simulink model "ROMmodel_C4_C5"  (R2023a)
settings: Solver=ode15s  StartTime=0.0  StopTime=1  RelTol=1e-3
model workspace (MAT data in the model file):
  AbdFlex : object (value not decoded)
  C_IVD = 0.05
  E_AbdFlex = 0.126
  E_IVD1 = 2.1
  E_IVD2 = 2.2
  E_IVD3 = 1.1
  E_IVD4 = 1.7
  E_IVD5 = 3.2
  E_IVD_Optimization : sldodialogs.data.SessionData (value not decoded)
  E_TLFflex = 18.8
  FollowerLoad = 0
  IAP_Pa = 0
  IAP_activation_AbdFlex = 1
  ISL_color = [1 1 1]
  ITL_color = [1 1 1]
  IVD1 : object (value not decoded)
  IVD2 : object (value not decoded)
  IVD3 : object (value not decoded)
  IVD4 : object (value not decoded)
  IVD5 : object (value not decoded)
  Left_EO = 0
  Left_IO = 0
  Left_Lat = 18
  Left_LongThor = 0
  Left_MF = 0
  Left_Psoas = 0
  Left_RA = 0
  Moment_AR = 0
  Moment_FE = 7.5
  Moment_LB = 0
  ROM_draft1_sdosession : sldodialogs.data.SessionData (value not decoded)
  ROM_draft3_sdosession : sldodialogs.data.SessionData (value not decoded)
  Right_EO = 0
  Right_IO = 0
  Right_Lat = 18
  Right_LongThor = 0
  Right_MF = 0
  Right_Psoas = 0
  Right_RA = 0
  SSL_color = [1 1 1]
  TLFflex : object (value not decoded)
  TLFflex2 : object (value not decoded)
  eo_color = [1 0 0]
  io_color = [0 1 0]
  latissimus_color = [0 0.5 1]
  ligament_radius = 0.5
  longissimus_color = [1 0 1]
  multifidus_color = [0 1 1]
  muscle_radius = 1
  psoas_color = [0 0 1]
  ra_color = [1 1 0.5]
libraries: checkpacklib x11, simulink_extras x9, simulink x2
settings Model: Creator=siril  LastModifiedBy=siril
settings DataIOCC: SaveFormat=StructureWithTime  ReturnWorkspaceOutputsName=SDOSimTest_Log
settings DebuggingCC: UniqueDataStoreMsg=error
settings SimscapeCC: SimscapeLogType=all

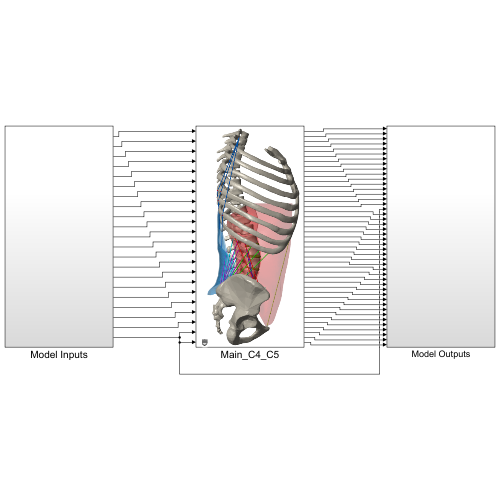
[thumbnail: Simulink's stored preview of the top-level diagram]
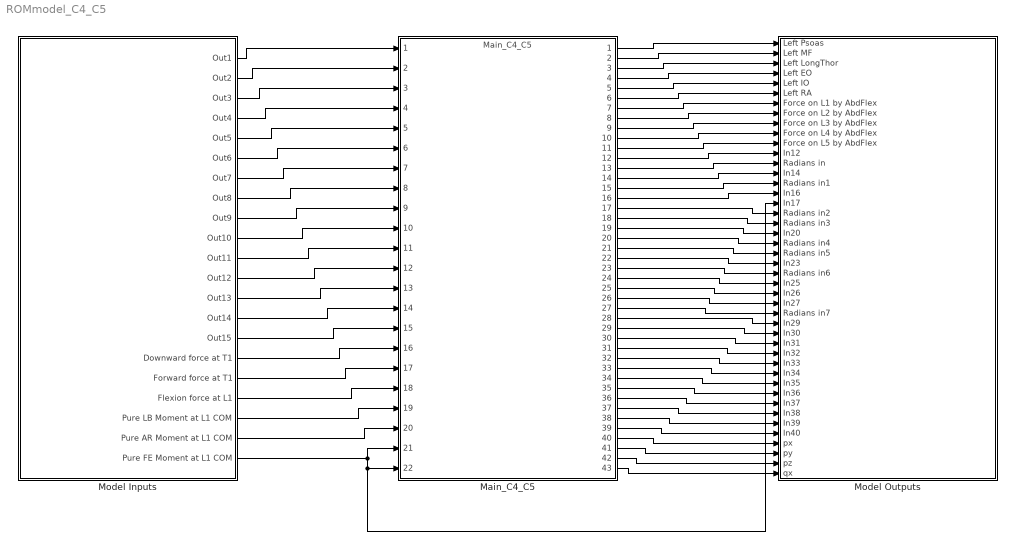
== system ROMmodel_C4_C5 ==
  Main_C4_C5 : ModelReference  ModelNameDialog=Main_C4_C5.slxp  ModelReferenceVersion=1.133  CopyOfModelProtected=on
      mask Display: image('$imagefile'); % Use "Edit Image" on toolstrip to change.
  Model Inputs : Subsystem
  Model Outputs : Subsystem
  wires:
  Main_C4_C5/32 -> Model Outputs/33(In33)
  Main_C4_C5/31 -> Model Outputs/32(In32)
  Model Inputs/20(Pure AR Moment at L1 COM) -> Main_C4_C5/20
  Model Inputs/1(Out1) -> Main_C4_C5/1
  Model Inputs/7(Out7) -> Main_C4_C5/7
  Model Inputs/8(Out8) -> Main_C4_C5/8
  Model Inputs/3(Out3) -> Main_C4_C5/3
  Main_C4_C5/20 -> Model Outputs/21(Radians in4)
  Model Inputs/2(Out2) -> Main_C4_C5/2
  Main_C4_C5/24 -> Model Outputs/25(In25)
  Main_C4_C5/23 -> Model Outputs/24(Radians in6)
  Model Inputs/12(Out12) -> Main_C4_C5/12
  Model Inputs/4(Out4) -> Main_C4_C5/4
  Main_C4_C5/13 -> Model Outputs/13(Radians in)
  Model Inputs/5(Out5) -> Main_C4_C5/5
  Model Inputs/6(Out6) -> Main_C4_C5/6
  Model Inputs/9(Out9) -> Main_C4_C5/9
  Main_C4_C5/19 -> Model Outputs/20(In20)
  Model Inputs/10(Out10) -> Main_C4_C5/10
  Main_C4_C5/22 -> Model Outputs/23(In23)
  Model Inputs/11(Out11) -> Main_C4_C5/11
  Main_C4_C5/25 -> Model Outputs/26(In26)
  Model Inputs/14(Out14) -> Main_C4_C5/14
  Model Inputs/13(Out13) -> Main_C4_C5/13
  Main_C4_C5/26 -> Model Outputs/27(In27)
  Model Inputs/15(Out15) -> Main_C4_C5/15
  Main_C4_C5/28 -> Model Outputs/29(In29)
  Main_C4_C5/27 -> Model Outputs/28(Radians in7)
  Model Inputs/16(Downward force at T1) -> Main_C4_C5/16
  Main_C4_C5/29 -> Model Outputs/30(In30)
  Model Inputs/18(Flexion force at L1) -> Main_C4_C5/18
  Model Inputs/17(Forward force at T1) -> Main_C4_C5/17
  Main_C4_C5/30 -> Model Outputs/31(In31)
  Model Inputs/19(Pure LB Moment at L1 COM) -> Main_C4_C5/19
  Main_C4_C5/33 -> Model Outputs/34(In34)
  Model Inputs/21(Pure FE Moment at L1 COM) -> Model Outputs/17(In17), Main_C4_C5/22, Main_C4_C5/21
  Main_C4_C5/1 -> Model Outputs/1(Left Psoas)
  Main_C4_C5/2 -> Model Outputs/2(Left MF)
  Main_C4_C5/3 -> Model Outputs/3(Left LongThor)
  Main_C4_C5/4 -> Model Outputs/4(Left EO)
  Main_C4_C5/5 -> Model Outputs/5(Left IO)
  Main_C4_C5/6 -> Model Outputs/6(Left RA)
  Main_C4_C5/7 -> Model Outputs/7(Force on L1 by AbdFlex)
  Main_C4_C5/8 -> Model Outputs/8(Force on L2 by AbdFlex)
  Main_C4_C5/9 -> Model Outputs/9(Force on L3 by AbdFlex)
  Main_C4_C5/10 -> Model Outputs/10(Force on L4 by AbdFlex)
  Main_C4_C5/11 -> Model Outputs/11(Force on L5 by AbdFlex)
  Main_C4_C5/12 -> Model Outputs/12(In12)
  Main_C4_C5/14 -> Model Outputs/14(In14)
  Main_C4_C5/15 -> Model Outputs/15(Radians in1)
  Main_C4_C5/16 -> Model Outputs/16(In16)
  Main_C4_C5/17 -> Model Outputs/18(Radians in2)
  Main_C4_C5/18 -> Model Outputs/19(Radians in3)
  Main_C4_C5/21 -> Model Outputs/22(Radians in5)
  Main_C4_C5/34 -> Model Outputs/35(In35)
  Main_C4_C5/36 -> Model Outputs/37(In37)
  Main_C4_C5/35 -> Model Outputs/36(In36)
  Main_C4_C5/37 -> Model Outputs/38(In38)
  Main_C4_C5/38 -> Model Outputs/39(In39)
  Main_C4_C5/40 -> Model Outputs/41(px)
  Main_C4_C5/39 -> Model Outputs/40(In40)
  Main_C4_C5/41 -> Model Outputs/42(py)
  Main_C4_C5/42 -> Model Outputs/43(pz)
  Main_C4_C5/43 -> Model Outputs/44(qx)

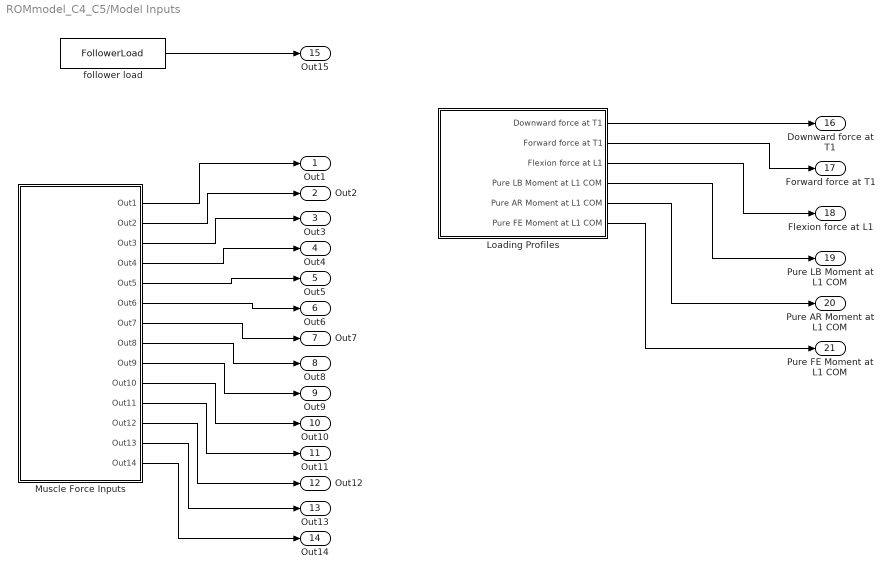
== system ROMmodel_C4_C5/Model Inputs ==
  Loading Profiles : Subsystem
  Muscle Force Inputs : Subsystem
  follower load : Constant  Value=FollowerLoad
  Out1 : Outport
  Out2 : Outport  Port=2
  Out3 : Outport  Port=3
  Out4 : Outport  Port=4
  Out5 : Outport  Port=5
  Out6 : Outport  Port=6
  Out7 : Outport  Port=7
  Out8 : Outport  Port=8
  Out9 : Outport  Port=9
  Out10 : Outport  Port=10
  Out11 : Outport  Port=11
  Out12 : Outport  Port=12
  Out13 : Outport  Port=13
  Out14 : Outport  Port=14
  Out15 : Outport  Port=15
  Downward force at T1 : Outport  Port=16
  Forward force at T1 : Outport  Port=17
  Flexion force at L1 : Outport  Port=18
  Pure LB Moment at L1 COM : Outport  Port=19
  Pure AR Moment at L1 COM : Outport  Port=20
  Pure FE Moment at L1 COM : Outport  Port=21
  wires:
  Muscle Force Inputs/12(Out12) -> Out12/1
  follower load/1 -> Out15/1
  Loading Profiles/6(Pure FE Moment at L1 COM) -> Pure FE Moment at L1 COM/1
  Loading Profiles/3(Flexion force at L1) -> Flexion force at L1/1
  Muscle Force Inputs/1(Out1) -> Out1/1
  Muscle Force Inputs/2(Out2) -> Out2/1
  Muscle Force Inputs/5(Out5) -> Out5/1
  Muscle Force Inputs/6(Out6) -> Out6/1
  Muscle Force Inputs/3(Out3) -> Out3/1
  Muscle Force Inputs/4(Out4) -> Out4/1
  Muscle Force Inputs/7(Out7) -> Out7/1
  Muscle Force Inputs/8(Out8) -> Out8/1
  Muscle Force Inputs/10(Out10) -> Out10/1
  Muscle Force Inputs/9(Out9) -> Out9/1
  Loading Profiles/4(Pure LB Moment at L1 COM) -> Pure LB Moment at L1 COM/1
  Muscle Force Inputs/11(Out11) -> Out11/1
  Muscle Force Inputs/13(Out13) -> Out13/1
  Muscle Force Inputs/14(Out14) -> Out14/1
  Loading Profiles/1(Downward force at T1) -> Downward force at T1/1
  Loading Profiles/2(Forward force at T1) -> Forward force at T1/1
  Loading Profiles/5(Pure AR Moment at L1 COM) -> Pure AR Moment at L1 COM/1

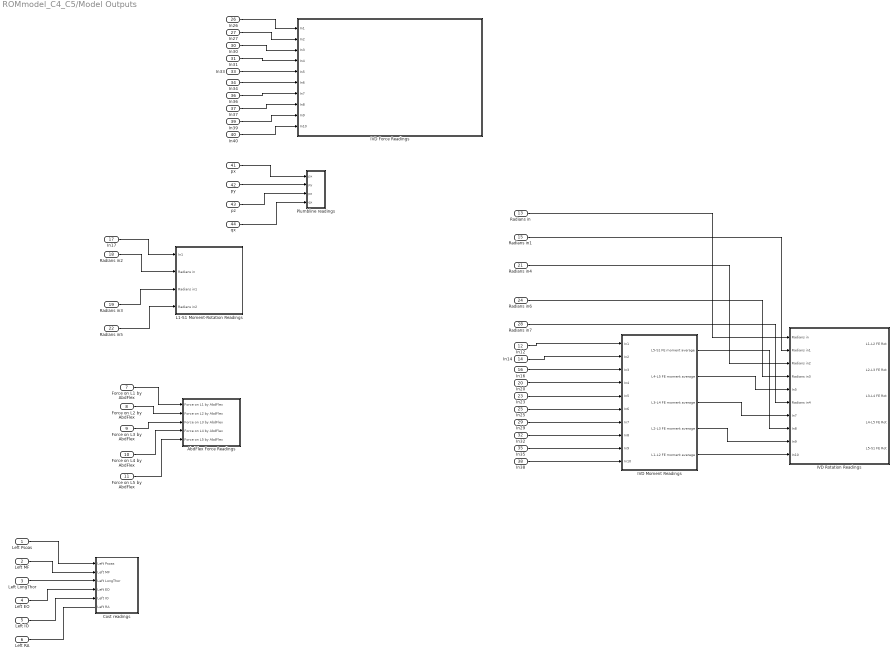
== system ROMmodel_C4_C5/Model Outputs ==
  Left Psoas : Inport
  Left MF : Inport  Port=2
  Left LongThor : Inport  Port=3
  Left EO : Inport  Port=4
  Left IO : Inport  Port=5
  Left RA : Inport  Port=6
  Force on L1 by AbdFlex : Inport  Port=7
  Force on L2 by AbdFlex : Inport  Port=8
  Force on L3 by AbdFlex : Inport  Port=9
  Force on L4 by AbdFlex : Inport  Port=10
  Force on L5 by AbdFlex : Inport  Port=11
  In12 : Inport  Port=12
  Radians in : Inport  Port=13
  In14 : Inport  Port=14
  Radians in1 : Inport  Port=15
  In16 : Inport  Port=16
  In17 : Inport  Port=17
  Radians in2 : Inport  Port=18
  Radians in3 : Inport  Port=19
  In20 : Inport  Port=20
  Radians in4 : Inport  Port=21
  Radians in5 : Inport  Port=22
  In23 : Inport  Port=23
  Radians in6 : Inport  Port=24
  In25 : Inport  Port=25
  In26 : Inport  Port=26
  In27 : Inport  Port=27
  Radians in7 : Inport  Port=28
  In29 : Inport  Port=29
  In30 : Inport  Port=30
  In31 : Inport  Port=31
  In32 : Inport  Port=32
  In33 : Inport  Port=33
  In34 : Inport  Port=34
  In35 : Inport  Port=35
  In36 : Inport  Port=36
  In37 : Inport  Port=37
  In38 : Inport  Port=38
  In39 : Inport  Port=39
  In40 : Inport  Port=40
  px : Inport  Port=41
  py : Inport  Port=42
  pz : Inport  Port=43
  qx : Inport  Port=44
  AbdFlex Force Readings : Subsystem
  Cost readings : Subsystem
  IVD Force Readings : Subsystem
  IVD Moment Readings : Subsystem
  IVD Rotation Readings : Subsystem
  L1-S1 Moment-Rotation Readings : Subsystem
  Plumbline readings : Subsystem
  wires:
  Left MF/1 -> Cost readings/2(Left MF)
  In37/1 -> IVD Force Readings/8(In8)
  In26/1 -> IVD Force Readings/1(In1)
  Radians in7/1 -> IVD Rotation Readings/6(Radians in4)
  In27/1 -> IVD Force Readings/2(In2)
  In30/1 -> IVD Force Readings/3(In3)
  In32/1 -> IVD Moment Readings/8(In8)
  In31/1 -> IVD Force Readings/4(In4)
  In33/1 -> IVD Force Readings/5(In5)
  In34/1 -> IVD Force Readings/6(In6)
  In35/1 -> IVD Moment Readings/9(In9)
  In36/1 -> IVD Force Readings/7(In7)
  Left EO/1 -> Cost readings/4(Left EO)
  In40/1 -> IVD Force Readings/10(In10)
  In39/1 -> IVD Force Readings/9(In9)
  px/1 -> Plumbline readings/1(px)
  py/1 -> Plumbline readings/2(py)
  qx/1 -> Plumbline readings/4(qx)
  pz/1 -> Plumbline readings/3(pz)
  Left Psoas/1 -> Cost readings/1(Left Psoas)
  Left LongThor/1 -> Cost readings/3(Left LongThor)
  Left IO/1 -> Cost readings/5(Left IO)
  Force on L1 by AbdFlex/1 -> AbdFlex Force Readings/1(Force on L1 by AbdFlex)
  Left RA/1 -> Cost readings/6(Left RA)
  In12/1 -> IVD Moment Readings/1(In1)
  Radians in1/1 -> IVD Rotation Readings/2(Radians in1)
  In14/1 -> IVD Moment Readings/2(In2)
  In16/1 -> IVD Moment Readings/3(In3)
  In20/1 -> IVD Moment Readings/4(In4)
  Radians in5/1 -> L1-S1 Moment-Rotation Readings/4(Radians in2)
  In23/1 -> IVD Moment Readings/5(In5)
  In25/1 -> IVD Moment Readings/6(In6)
  In29/1 -> IVD Moment Readings/7(In7)
  In38/1 -> IVD Moment Readings/10(In10)
  IVD Moment Readings/1(L5-S1 FE moment average) -> IVD Rotation Readings/8(In8)
  Radians in/1 -> IVD Rotation Readings/1(Radians in)
  In17/1 -> L1-S1 Moment-Rotation Readings/1(In1)
  Radians in3/1 -> L1-S1 Moment-Rotation Readings/3(Radians in1)
  Radians in2/1 -> L1-S1 Moment-Rotation Readings/2(Radians in)
  Radians in4/1 -> IVD Rotation Readings/3(Radians in2)
  Radians in6/1 -> IVD Rotation Readings/4(Radians in3)
  Force on L2 by AbdFlex/1 -> AbdFlex Force Readings/2(Force on L2 by AbdFlex)
  Force on L3 by AbdFlex/1 -> AbdFlex Force Readings/3(Force on L3 by AbdFlex)
  Force on L5 by AbdFlex/1 -> AbdFlex Force Readings/5(Force on L5 by AbdFlex)
  Force on L4 by AbdFlex/1 -> AbdFlex Force Readings/4(Force on L4 by AbdFlex)
  IVD Moment Readings/2(L4-L5 FE moment average) -> IVD Rotation Readings/5(In5)
  IVD Moment Readings/3(L3-L4 FE moment average) -> IVD Rotation Readings/7(In7)
  IVD Moment Readings/4(L2-L3 FE moment average) -> IVD Rotation Readings/9(In9)
  IVD Moment Readings/5(L1-L2 FE moment average) -> IVD Rotation Readings/10(In10)

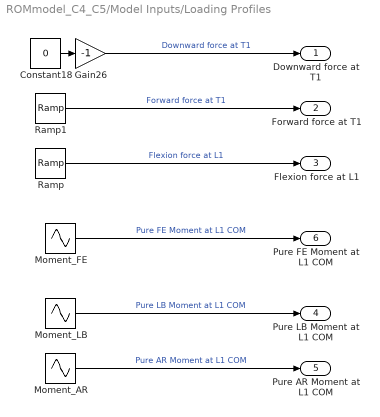
== system ROMmodel_C4_C5/Model Inputs/Loading Profiles ==
  Constant18 : Constant  Value=0
  Gain26 : Gain  Gain=-1
  Moment_AR : Sin  Amplitude=Moment_AR  Frequency=pi*0.5  SampleTime=0
  Moment_FE : Sin  Amplitude=-Moment_FE  Frequency=pi*0.5  SampleTime=0
  Moment_LB : Sin  Amplitude=-Moment_LB  Frequency=pi*0.5  SampleTime=0
  Ramp : simulink/Sources/Ramp  slope=0  start=0  InitialOutput=0
  Ramp1 : simulink/Sources/Ramp  slope=0  start=0  InitialOutput=0
  Downward force at T1 : Outport
  Forward force at T1 : Outport  Port=2
  Flexion force at L1 : Outport  Port=3
  Pure LB Moment at L1 COM : Outport  Port=4
  Pure AR Moment at L1 COM : Outport  Port=5
  Pure FE Moment at L1 COM : Outport  Port=6
  wires:
  Ramp1/1 -> Forward force at T1/1  "Forward force at T1"
  Moment_AR/1 -> Pure AR Moment at L1 COM/1  "Pure AR Moment at L1 COM"
  Constant18/1 -> Gain26/1
  Ramp/1 -> Flexion force at L1/1  "Flexion force at L1"
  Moment_LB/1 -> Pure LB Moment at L1 COM/1  "Pure LB Moment at L1 COM"
  Moment_FE/1 -> Pure FE Moment at L1 COM/1  "Pure FE Moment at L1 COM"
  Gain26/1 -> Downward force at T1/1  "Downward force at T1"

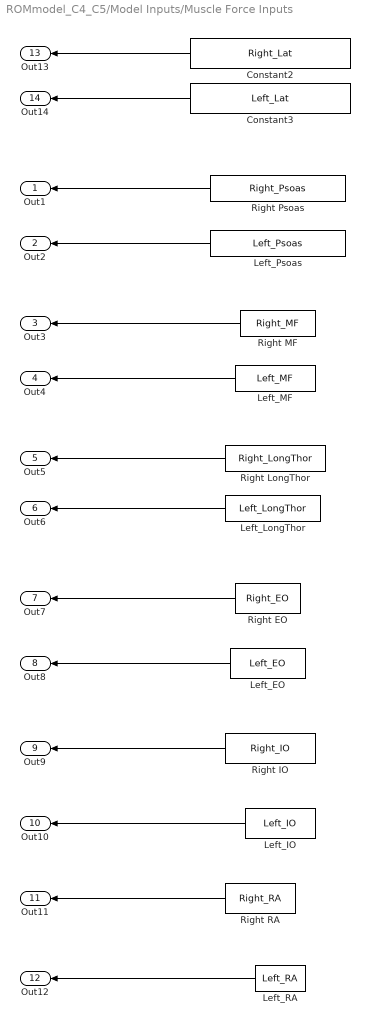
== system ROMmodel_C4_C5/Model Inputs/Muscle Force Inputs ==
  Constant2 : Constant  Value=Right_Lat
  Constant3 : Constant  Value=Left_Lat
  Left_EO : Constant  Value=Left_EO
  Left_IO : Constant  Value=Left_IO
  Left_LongThor : Constant  Value=Left_LongThor
  Left_MF : Constant  Value=Left_MF
  Left_Psoas : Constant  Value=Left_Psoas
  Left_RA : Constant  Value=Left_RA
  Right EO : Constant  Value=Right_EO
  Right IO : Constant  Value=Right_IO
  Right LongThor : Constant  Value=Right_LongThor
  Right MF : Constant  Value=Right_MF
  Right Psoas : Constant  Value=Right_Psoas
  Right RA : Constant  Value=Right_RA
  Out1 : Outport
  Out2 : Outport  Port=2
  Out3 : Outport  Port=3
  Out4 : Outport  Port=4
  Out5 : Outport  Port=5
  Out6 : Outport  Port=6
  Out7 : Outport  Port=7
  Out8 : Outport  Port=8
  Out9 : Outport  Port=9
  Out10 : Outport  Port=10
  Out11 : Outport  Port=11
  Out12 : Outport  Port=12
  Out13 : Outport  Port=13
  Out14 : Outport  Port=14
  wires:
  Right MF/1 -> Out3/1
  Right IO/1 -> Out9/1
  Left_EO/1 -> Out8/1
  Constant3/1 -> Out14/1
  Constant2/1 -> Out13/1
  Right EO/1 -> Out7/1
  Right LongThor/1 -> Out5/1
  Left_LongThor/1 -> Out6/1
  Right Psoas/1 -> Out1/1
  Left_RA/1 -> Out12/1
  Left_IO/1 -> Out10/1
  Right RA/1 -> Out11/1
  Left_MF/1 -> Out4/1
  Left_Psoas/1 -> Out2/1

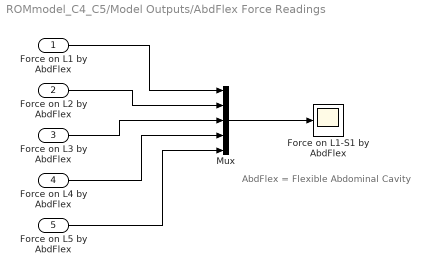
== system ROMmodel_C4_C5/Model Outputs/AbdFlex Force Readings ==
  note: AbdFlex = Flexible Abdominal Cavity
  Force on L1 by AbdFlex : Inport
  Force on L2 by AbdFlex : Inport  Port=2
  Force on L3 by AbdFlex : Inport  Port=3
  Force on L4 by AbdFlex : Inport  Port=4
  Force on L5 by AbdFlex : Inport  Port=5
  Force on L1-S1 by AbdFlex : Scope
  Mux : Mux  Inputs=5
  wires:
  Mux/1 -> Force on L1-S1 by AbdFlex/1
  Force on L4 by AbdFlex/1 -> Mux/4
  Force on L3 by AbdFlex/1 -> Mux/3
  Force on L5 by AbdFlex/1 -> Mux/5
  Force on L2 by AbdFlex/1 -> Mux/2
  Force on L1 by AbdFlex/1 -> Mux/1

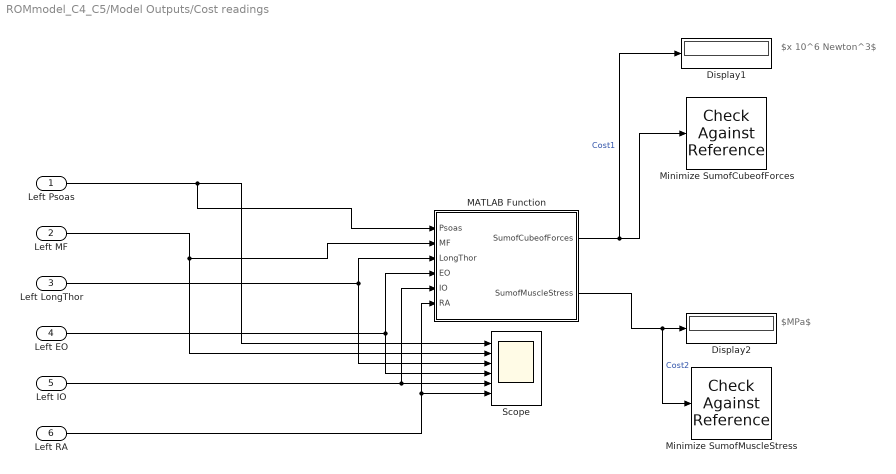
== system ROMmodel_C4_C5/Model Outputs/Cost readings ==
  note: $x 10^6 Newton^3$
  note: $MPa$
  Left Psoas : Inport
  Left MF : Inport  Port=2
  Left LongThor : Inport  Port=3
  Left EO : Inport  Port=4
  Left IO : Inport  Port=5
  Left RA : Inport  Port=6
  Display1 : Display
  Display2 : Display
  MATLAB Function : MATLAB Function
  Minimize SumofCubeofForces : checkpacklib/Check Against Reference  InstantiateOnLoad=on  enabled=off  stopWhenAssertionFail=off  export=off  ViewDlgPos=[360 309 510 390]  OpenViewOnLoad=off  LaunchViewOnOpen=off  TimeUnits=seconds  EnableReferenceBound=on  AbsTolerance=0  RelTolerance=0  ReferenceTimes=[0 1]  ReferenceAmplitudes=[0 0];
      settings: LibrarySourceBlock=sdolib/Signal Constraints/Check Against Reference
  Minimize SumofMuscleStress : checkpacklib/Check Against Reference  InstantiateOnLoad=on  enabled=off  stopWhenAssertionFail=off  export=off  ViewDlgPos=[360 309 510 390]  OpenViewOnLoad=off  LaunchViewOnOpen=off  TimeUnits=seconds  EnableReferenceBound=on  AbsTolerance=0  RelTolerance=0  ReferenceTimes=[0 1]  ReferenceAmplitudes=[0 0];
      settings: LibrarySourceBlock=sdolib/Signal Constraints/Check Against Reference
  Scope : Scope  NumInputPorts=6
  wires:
  MATLAB Function/2(SumofMuscleStress) -> Minimize SumofMuscleStress/1, Display2/1  "Cost2"
  Left EO/1 -> Scope/4, MATLAB Function/4(EO)
  Left RA/1 -> Scope/6, MATLAB Function/6(RA)
  Left IO/1 -> Scope/5, MATLAB Function/5(IO)
  Left MF/1 -> MATLAB Function/2(MF), Scope/2
  Left Psoas/1 -> MATLAB Function/1(Psoas), Scope/1
  Left LongThor/1 -> Scope/3, MATLAB Function/3(LongThor)
  MATLAB Function/1(SumofCubeofForces) -> Minimize SumofCubeofForces/1, Display1/1  "Cost1"

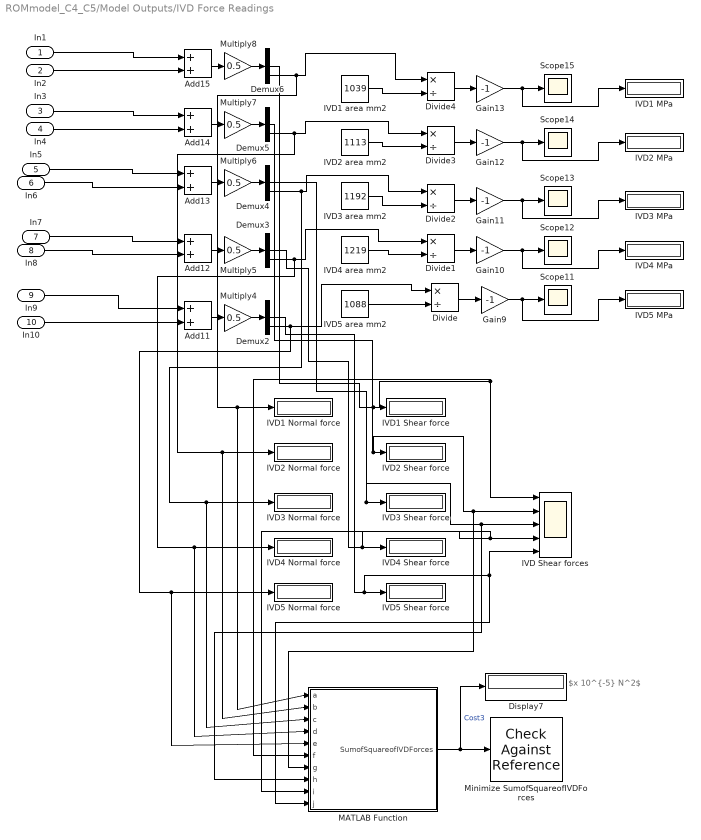
== system ROMmodel_C4_C5/Model Outputs/IVD Force Readings ==
  note: $x 10^{-5} N^2$
  In1 : Inport
  In2 : Inport  Port=2
  In3 : Inport  Port=3
  In4 : Inport  Port=4
  In5 : Inport  Port=5
  In6 : Inport  Port=6
  In7 : Inport  Port=7
  In8 : Inport  Port=8
  In9 : Inport  Port=9
  In10 : Inport  Port=10
  Add{11..15} (5 alike) : Sum
  Demux{2..6} (5 alike) : Demux  Outputs=3
  Display7 : Display
  Divide{,1..4} (5 alike) : Product  Inputs=*/
  Gain{9..13} (5 alike) : Gain  Gain=-1
  IVD Shear forces : Scope  NumInputPorts=5
  IVD1 MPa : Display
  IVD1 Normal force : Display
  IVD1 Shear force : Display
  IVD1 area mm2 : Constant  Value=1039
  IVD2 MPa : Display
  IVD2 Normal force : Display
  IVD2 Shear force : Display
  IVD2 area mm2 : Constant  Value=1113
  IVD3 MPa : Display
  IVD3 Normal force : Display
  IVD3 Shear force : Display
  IVD3 area mm2 : Constant  Value=1192
  IVD4 MPa : Display
  IVD4 Normal force : Display
  IVD4 Shear force : Display
  IVD4 area mm2 : Constant  Value=1219
  IVD5 MPa : Display
  IVD5 Normal force : Display
  IVD5 Shear force : Display
  IVD5 area mm2 : Constant  Value=1088
  MATLAB Function : MATLAB Function  TreatAsAtomicUnit=on
  Minimize SumofSquareofIVDForces : checkpacklib/Check Against Reference  InstantiateOnLoad=on  enabled=off  stopWhenAssertionFail=off  export=off  ViewDlgPos=[360 309 510 390]  OpenViewOnLoad=off  LaunchViewOnOpen=off  TimeUnits=seconds  EnableReferenceBound=on  AbsTolerance=0  RelTolerance=0  ReferenceTimes=[0 1]  ReferenceAmplitudes=[0 0];
      settings: LibrarySourceBlock=sdolib/Signal Constraints/Check Against Reference
  Multiply{4..8} (5 alike) : Gain  Gain=0.5
  Scope{11..15} (5 alike) : Scope
  wires:
  Gain12/1 -> IVD2 MPa/1, Scope14/1
  Multiply6/1 -> Demux4/1
  Demux2/3 -> MATLAB Function/5(e), IVD5 Normal force/1, Divide/1
  Demux3/2 -> MATLAB Function/9(i), IVD Shear forces/4, IVD4 Shear force/1
  Demux5/3 -> MATLAB Function/2(b), IVD2 Normal force/1, Divide3/1
  Add13/1 -> Multiply6/1
  Multiply7/1 -> Demux5/1
  Divide1/1 -> Gain10/1
  Demux5/2 -> IVD Shear forces/2, MATLAB Function/7(g), IVD2 Shear force/1
  IVD4 area mm2/1 -> Divide1/2
  Add14/1 -> Multiply7/1
  In7/1 -> Add12/1
  IVD2 area mm2/1 -> Divide3/2
  IVD3 area mm2/1 -> Divide2/2
  Demux4/3 -> MATLAB Function/3(c), IVD3 Normal force/1, Divide2/1
  Multiply4/1 -> Demux2/1
  Demux6/2 -> IVD Shear forces/1, MATLAB Function/6(f), IVD1 Shear force/1
  Gain10/1 -> IVD4 MPa/1, Scope12/1
  IVD1 area mm2/1 -> Divide4/2
  IVD5 area mm2/1 -> Divide/2
  Multiply8/1 -> Demux6/1
  Add12/1 -> Multiply5/1
  Add15/1 -> Multiply8/1
  Divide/1 -> Gain9/1
  Gain11/1 -> IVD3 MPa/1, Scope13/1
  Demux4/2 -> IVD Shear forces/3, MATLAB Function/8(h), IVD3 Shear force/1
  Multiply5/1 -> Demux3/1
  Divide2/1 -> Gain11/1
  Divide3/1 -> Gain12/1
  MATLAB Function/1(SumofSquareofIVDForces) -> Minimize SumofSquareofIVDForces/1, Display7/1  "Cost3"
  Demux3/3 -> MATLAB Function/4(d), IVD4 Normal force/1, Divide1/1
  In10/1 -> Add11/2
  Demux6/3 -> MATLAB Function/1(a), IVD1 Normal force/1, Divide4/1
  Demux2/2 -> IVD Shear forces/5, MATLAB Function/10(j), IVD5 Shear force/1
  In5/1 -> Add13/1
  Divide4/1 -> Gain13/1
  Gain9/1 -> IVD5 MPa/1, Scope11/1
  In9/1 -> Add11/1
  Add11/1 -> Multiply4/1
  Gain13/1 -> IVD1 MPa/1, Scope15/1
  In3/1 -> Add14/1
  In4/1 -> Add14/2
  In6/1 -> Add13/2
  In1/1 -> Add15/1
  In8/1 -> Add12/2
  In2/1 -> Add15/2

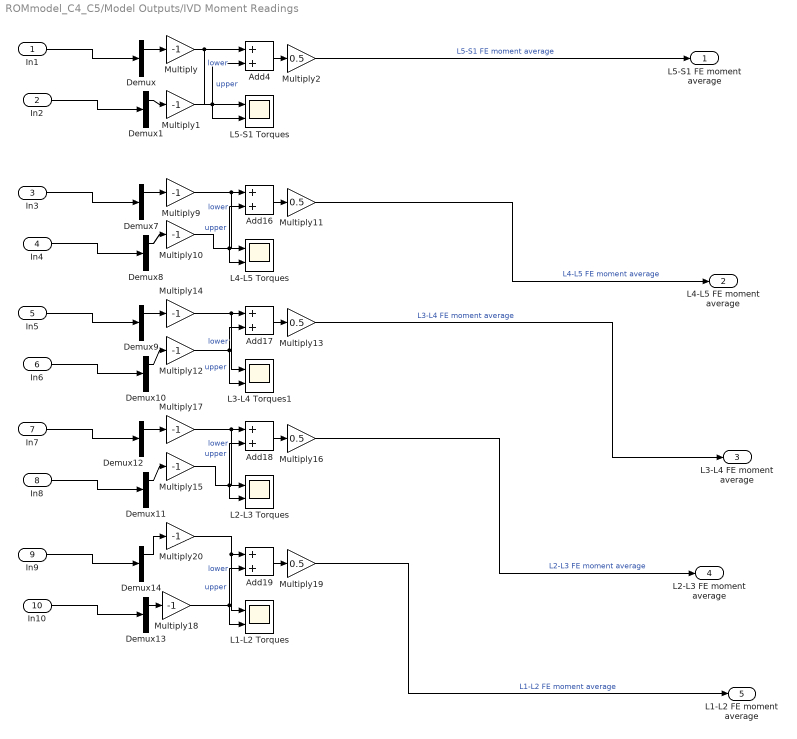
== system ROMmodel_C4_C5/Model Outputs/IVD Moment Readings ==
  In1 : Inport
  In2 : Inport  Port=2
  In3 : Inport  Port=3
  In4 : Inport  Port=4
  In5 : Inport  Port=5
  In6 : Inport  Port=6
  In7 : Inport  Port=7
  In8 : Inport  Port=8
  In9 : Inport  Port=9
  In10 : Inport  Port=10
  Add{4,16..19} (5 alike) : Sum
  Demux{,1,7..14} (10 alike) : Demux  Outputs=3
  L1-L2 Torques : Scope  NumInputPorts=2
  L2-L3 Torques : Scope  NumInputPorts=2
  L3-L4 Torques1 : Scope  NumInputPorts=2
  L4-L5 Torques : Scope  NumInputPorts=2
  L5-S1 Torques : Scope  NumInputPorts=2
  Multiply{,1,9..10,12,14..15,17..18,20} (10 alike) : Gain  Gain=-1
  Multiply{2,11,13,16,19} (5 alike) : Gain  Gain=0.5
  L5-S1 FE moment average : Outport
  L4-L5 FE moment average : Outport  Port=2
  L3-L4 FE moment average : Outport  Port=3
  L2-L3 FE moment average : Outport  Port=4
  L1-L2 FE moment average : Outport  Port=5
  wires:
  Multiply13/1 -> L3-L4 FE moment average/1  "L3-L4 FE moment average"
  Multiply18/1 -> L1-L2 Torques/2, Add19/2  "upper"
  In5/1 -> Demux9/1
  Multiply15/1 -> L2-L3 Torques/2, Add18/2  "upper"
  Add16/1 -> Multiply11/1
  Multiply1/1 -> L5-S1 Torques/2, Add4/2  "upper"
  In4/1 -> Demux8/1
  Multiply20/1 -> Add19/1, L1-L2 Torques/1  "lower"
  Multiply10/1 -> L4-L5 Torques/2, Add16/2  "upper"
  Multiply12/1 -> L3-L4 Torques1/2, Add17/2  "upper"
  Add18/1 -> Multiply16/1
  Multiply2/1 -> L5-S1 FE moment average/1  "L5-S1 FE moment average"
  Add17/1 -> Multiply13/1
  Demux12/1 -> Multiply17/1
  Multiply17/1 -> Add18/1, L2-L3 Torques/1  "lower"
  Demux1/1 -> Multiply1/1
  Demux10/1 -> Multiply12/1
  Demux14/1 -> Multiply20/1
  Multiply16/1 -> L2-L3 FE moment average/1  "L2-L3 FE moment average"
  Demux9/1 -> Multiply14/1
  Add19/1 -> Multiply19/1
  Multiply14/1 -> Add17/1, L3-L4 Torques1/1  "lower"
  Multiply9/1 -> Add16/1, L4-L5 Torques/1  "lower"
  In2/1 -> Demux1/1
  In7/1 -> Demux12/1
  In3/1 -> Demux7/1
  Multiply/1 -> Add4/1, L5-S1 Torques/1  "lower"
  Add4/1 -> Multiply2/1
  In1/1 -> Demux/1
  Demux/1 -> Multiply/1
  Demux11/1 -> Multiply15/1
  Demux8/1 -> Multiply10/1
  Demux13/1 -> Multiply18/1
  In8/1 -> Demux11/1
  Demux7/1 -> Multiply9/1
  Multiply19/1 -> L1-L2 FE moment average/1  "L1-L2 FE moment average"
  Multiply11/1 -> L4-L5 FE moment average/1  "L4-L5 FE moment average"
  In6/1 -> Demux10/1
  In9/1 -> Demux14/1
  In10/1 -> Demux13/1

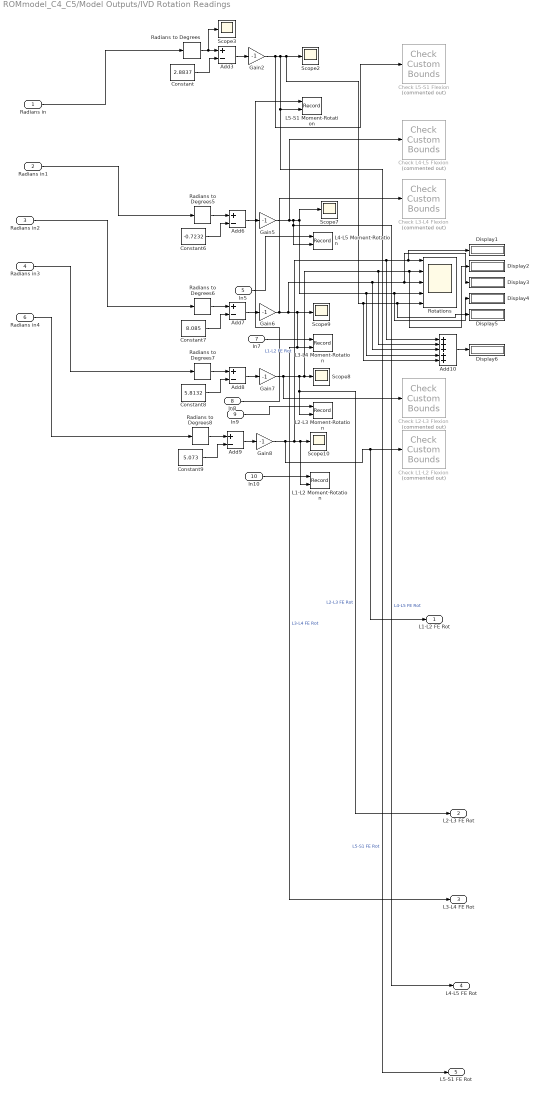
== system ROMmodel_C4_C5/Model Outputs/IVD Rotation Readings ==
  Radians in : Inport
  Radians in1 : Inport  Port=2
  Radians in2 : Inport  Port=3
  Radians in3 : Inport  Port=4
  In5 : Inport  Port=5
  Radians in4 : Inport  Port=6
  In7 : Inport  Port=7
  In8 : Inport  Port=8
  In9 : Inport  Port=9
  In10 : Inport  Port=10
  Add10 : Sum  Inputs=+++++
  Add{3,6..9} (5 alike) : Sum  Inputs=+-
  Check L1-L2 Flexion : checkpacklib/Check Custom Bounds  Commented=on  InstantiateOnLoad=on  enabled=off  stopWhenAssertionFail=off  export=off  ViewDlgPos=[697 199.4 508.8 390.4]  OpenViewOnLoad=off  LaunchViewOnOpen=off  TimeUnits=seconds  EnableUpperBound=on  UpperBoundTimes=[0.54 0.56]  UpperBoundAmplitudes=[10.8 10.8]  EnableLowerBound=on  LowerBoundTimes=[0.54 0.56]  LowerBoundAmplitudes=[6.8 6.8]
      settings: LibrarySourceBlock=sdolib/Signal Constraints/Check Custom Bounds
  Check L2-L3 Flexion : checkpacklib/Check Custom Bounds  Commented=on  InstantiateOnLoad=on  enabled=off  stopWhenAssertionFail=off  export=off  ViewDlgPos=[1119 206 510 390]  OpenViewOnLoad=off  LaunchViewOnOpen=off  TimeUnits=seconds  EnableUpperBound=on  UpperBoundTimes=[0.54 0.56]  UpperBoundAmplitudes=[9.9 9.9]  EnableLowerBound=on  LowerBoundTimes=[0.54 0.56]  LowerBoundAmplitudes=[7.3 7.3]
      settings: LibrarySourceBlock=sdolib/Signal Constraints/Check Custom Bounds
  Check L3-L4 Flexion : checkpacklib/Check Custom Bounds  Commented=on  InstantiateOnLoad=on  enabled=off  stopWhenAssertionFail=off  export=off  ViewDlgPos=[1119 206 510 390]  OpenViewOnLoad=off  LaunchViewOnOpen=off  TimeUnits=seconds  EnableUpperBound=on  UpperBoundTimes=[0.54 0.56]  UpperBoundAmplitudes=[8.8 8.8]  EnableLowerBound=on  LowerBoundTimes=[0.54 0.56]  LowerBoundAmplitudes=[6.4 6.4]
      settings: LibrarySourceBlock=sdolib/Signal Constraints/Check Custom Bounds
  Check L4-L5 Flexion : checkpacklib/Check Custom Bounds  Commented=on  InstantiateOnLoad=on  enabled=off  stopWhenAssertionFail=off  export=off  ViewDlgPos=[1119 206 510 390]  OpenViewOnLoad=off  LaunchViewOnOpen=off  TimeUnits=seconds  EnableUpperBound=on  UpperBoundTimes=[0.54 0.56]  UpperBoundAmplitudes=[7.1 7.1]  EnableLowerBound=on  LowerBoundTimes=[0.54 0.56]  LowerBoundAmplitudes=[4.3 4.3]
      settings: LibrarySourceBlock=sdolib/Signal Constraints/Check Custom Bounds
  Check L5-S1 Flexion : checkpacklib/Check Custom Bounds  Commented=on  InstantiateOnLoad=on  enabled=off  stopWhenAssertionFail=off  export=off  ViewDlgPos=[2337 185 510 390]  OpenViewOnLoad=off  LaunchViewOnOpen=off  TimeUnits=seconds  EnableUpperBound=on  UpperBoundTimes=[0.54 0.56]  UpperBoundAmplitudes=[4.7 4.7]  EnableLowerBound=on  LowerBoundTimes=[0.54 0.56]  LowerBoundAmplitudes=[2.7 2.7]
      settings: LibrarySourceBlock=sdolib/Signal Constraints/Check Custom Bounds
  Constant : Constant  Value=2.8837
  Constant6 : Constant  Value=-0.7232
  Constant7 : Constant  Value=8.085
  Constant8 : Constant  Value=5.8132
  Constant9 : Constant  Value=5.073
  Display{1..6} (6 alike) : Display
  Gain{2,5..8} (5 alike) : Gain  Gain=-1
  L1-L2 Moment-Rotation : Record  FileSettingsInternal="{\"entries\":[{\"content\":{\"excelSettings\":{\"content\":{},\"type\":\"Streamout.ExcelSettings\"}},\"type\":\"Streamout.FileSettingStorage\",\"uuid\":\"29a2f603-6b8f-42fc-9601-ae1617b0b55d\"}],\"packageUris\":[\"http://schema.mathworks.com/mf0/streamout_blocks/R2020a\"],\"version\":\"1.0\"}"  Layout=[1 1]  SignalsInternal="{\"entries\":[{\"content\":{\"signals\":[{\"content\":{\"blockPath\":[\"ROMmodel_C4_C5/Model Outputs/IVD Rotation Readings/L1-L2 Moment-Rotation\"],\"dimensions\":[1,1],\"domain\":\"ROMmodel_C4_C5/Model Outputs/IVD Rotation Readings/L1-L2 Moment-Rotation\",\"lineColor\":\"#0072bd\",\"port\":1,\"sid\":[\"\"],\"signalID\":71,\"signalName\":\"In10\"},\"type\":\"RecordBlkView.Signal\",\"uuid\":\"ffa4c73e-feb2-45f1-8c21-1bd0adc7f99c\"},{\"content\":{\"blockPath\":[\"ROMmodel_C4_C5/Model Outputs/IVD Rotation Readings/L1-L2 Moment-Rotation\"],\"dimensions\":[1,1],\"domain\":\"ROMmodel_C4_C5/Model Outputs/IVD Rotation Readings/L1-L2 Moment-Rotation\",\"lineColor\":\"#d95319\",\"port\":2,\"sid\":[\"\"],\"signalID\":75,\"signalName\":\"L1-L2 FE Rot\"},\"type\":\"RecordBlkView.Signal\",\"uuid\":\"ebf1cb2d-af00-4fce-b05f-50c524fbcd6e\"}]},\"type\":\"RecordBlkView.InputSignals\",\"uuid\":\"0633209f-fff6-4dbf-bc21-c7989228a926\"}],\"packageUris\":[\"http://schema.mathworks.com/mf0/streamout_blocks/R2020a\"],\"version\":\"1.0\"}"  VisualizationBindings="{\"xyplotplugin\":{\"info\":[{\"series\":[],\"subplotID\":1}]}}"  CompatibilityTag=XY
  L2-L3 Moment-Rotation : Record  FileSettingsInternal="{\"entries\":[{\"content\":{\"excelSettings\":{\"content\":{},\"type\":\"Streamout.ExcelSettings\"}},\"type\":\"Streamout.FileSettingStorage\",\"uuid\":\"1b2af98a-d10d-4ce5-8fa7-061aa41184de\"}],\"packageUris\":[\"http://schema.mathworks.com/mf0/streamout_blocks/R2020a\"],\"version\":\"1.0\"}"  Layout=[1 1]  SignalsInternal="{\"entries\":[{\"content\":{\"signals\":[{\"content\":{\"blockPath\":[\"ROMmodel_C4_C5/Model Outputs/IVD Rotation Readings/L2-L3 Moment-Rotation\"],\"dimensions\":[1,1],\"domain\":\"ROMmodel_C4_C5/Model Outputs/IVD Rotation Readings/L2-L3 Moment-Rotation\",\"lineColor\":\"#edb120\",\"port\":1,\"sid\":[\"\"],\"signalID\":79,\"signalName\":\"In9\"},\"type\":\"RecordBlkView.Signal\",\"uuid\":\"49fa3249-2b63-45e5-803d-7eca4a0bfae1\"},{\"content\":{\"blockPath\":[\"ROMmodel_C4_C5/Model Outputs/IVD Rotation Readings/L2-L3 Moment-Rotation\"],\"dimensions\":[1,1],\"domain\":\"ROMmodel_C4_C5/Model Outputs/IVD Rotation Readings/L2-L3 Moment-Rotation\",\"lineColor\":\"#7e2f8e\",\"port\":2,\"sid\":[\"\"],\"signalID\":83,\"signalName\":\"L2-L3 FE Rot\"},\"type\":\"RecordBlkView.Signal\",\"uuid\":\"1e2f28df-401c-4561-b152-f4ca42b91d51\"}]},\"type\":\"RecordBlkView.InputSignals\",\"uuid\":\"2cefbbf2-1aa9-4da4-a04b-fd53bc7ff3ec\"}],\"packageUris\":[\"http://schema.mathworks.com/mf0/streamout_blocks/R2020a\"],\"version\":\"1.0\"}"  VisualizationBindings="{\"xyplotplugin\":{\"info\":[{\"series\":[],\"subplotID\":1}]}}"  CompatibilityTag=XY
  L3-L4 Moment-Rotation : Record  FileSettingsInternal="{\"entries\":[{\"content\":{\"excelSettings\":{\"content\":{},\"type\":\"Streamout.ExcelSettings\"}},\"type\":\"Streamout.FileSettingStorage\",\"uuid\":\"97fd5132-f967-4270-b036-55d1c5563848\"}],\"packageUris\":[\"http://schema.mathworks.com/mf0/streamout_blocks/R2020a\"],\"version\":\"1.0\"}"  Layout=[1 1]  SignalsInternal="{\"entries\":[{\"content\":{\"signals\":[{\"content\":{\"blockPath\":[\"ROMmodel_C4_C5/Model Outputs/IVD Rotation Readings/L3-L4 Moment-Rotation\"],\"dimensions\":[1,1],\"domain\":\"ROMmodel_C4_C5/Model Outputs/IVD Rotation Readings/L3-L4 Moment-Rotation\",\"lineColor\":\"#77ac30\",\"port\":1,\"sid\":[\"\"],\"signalID\":87,\"signalName\":\"In7\"},\"type\":\"RecordBlkView.Signal\",\"uuid\":\"1cf7e802-00ab-4430-853a-9260ff5c8af9\"},{\"content\":{\"blockPath\":[\"ROMmodel_C4_C5/Model Outputs/IVD Rotation Readings/L3-L4 Moment-Rotation\"],\"dimensions\":[1,1],\"domain\":\"ROMmodel_C4_C5/Model Outputs/IVD Rotation Readings/L3-L4 Moment-Rotation\",\"lineColor\":\"#4dbeee\",\"port\":2,\"sid\":[\"\"],\"signalID\":91,\"signalName\":\"L3-L4 FE Rot\"},\"type\":\"RecordBlkView.Signal\",\"uuid\":\"53a9f570-cb14-47e1-9a43-3c019409e759\"}]},\"type\":\"RecordBlkView.InputSignals\",\"uuid\":\"e819156f-c4c8-4f4e-9d7e-445d87c6c61b\"}],\"packageUris\":[\"http://schema.mathworks.com/mf0/streamout_blocks/R2020a\"],\"version\":\"1.0\"}"  VisualizationBindings="{\"xyplotplugin\":{\"info\":[{\"series\":[],\"subplotID\":1}]}}"  CompatibilityTag=XY
  L4-L5 Moment-Rotation : Record  FileSettingsInternal="{\"entries\":[{\"content\":{\"excelSettings\":{\"content\":{},\"type\":\"Streamout.ExcelSettings\"}},\"type\":\"Streamout.FileSettingStorage\",\"uuid\":\"c68568d6-c550-4b60-9913-9b95521d7d31\"}],\"packageUris\":[\"http://schema.mathworks.com/mf0/streamout_blocks/R2020a\"],\"version\":\"1.0\"}"  Layout=[1 1]  SignalsInternal="{\"entries\":[{\"content\":{\"signals\":[{\"content\":{\"blockPath\":[\"ROMmodel_C4_C5/Model Outputs/IVD Rotation Readings/L4-L5 Moment-Rotation\"],\"dimensions\":[1,1],\"domain\":\"ROMmodel_C4_C5/Model Outputs/IVD Rotation Readings/L4-L5 Moment-Rotation\",\"lineColor\":\"#a2142f\",\"port\":1,\"sid\":[\"\"],\"signalID\":95,\"signalName\":\"In5\"},\"type\":\"RecordBlkView.Signal\",\"uuid\":\"b979d3c3-422f-4205-a9b0-9a90138a5a78\"},{\"content\":{\"blockPath\":[\"ROMmodel_C4_C5/Model Outputs/IVD Rotation Readings/L4-L5 Moment-Rotation\"],\"dimensions\":[1,1],\"domain\":\"ROMmodel_C4_C5/Model Outputs/IVD Rotation Readings/L4-L5 Moment-Rotation\",\"lineColor\":\"#139fff\",\"port\":2,\"sid\":[\"\"],\"signalID\":99,\"signalName\":\"L4-L5 FE Rot\"},\"type\":\"RecordBlkView.Signal\",\"uuid\":\"2850d68a-7a52-4050-af95-21616ac6f320\"}]},\"type\":\"RecordBlkView.InputSignals\",\"uuid\":\"f3fa91fd-96b6-4ac5-837c-f9ff340526e2\"}],\"packageUris\":[\"http://schema.mathworks.com/mf0/streamout_blocks/R2020a\"],\"version\":\"1.0\"}"  VisualizationBindings="{\"xyplotplugin\":{\"info\":[{\"series\":[],\"subplotID\":1}]}}"  CompatibilityTag=XY
  L5-S1 Moment-Rotation : Record  FileSettingsInternal="{\"entries\":[{\"content\":{\"excelSettings\":{\"content\":{},\"type\":\"Streamout.ExcelSettings\"}},\"type\":\"Streamout.FileSettingStorage\",\"uuid\":\"2eb4ad7a-8235-4702-8c6b-c0f0ba1a5dc9\"}],\"packageUris\":[\"http://schema.mathworks.com/mf0/streamout_blocks/R2020a\"],\"version\":\"1.0\"}"  Layout=[1 1]  SignalsInternal="{\"entries\":[{\"content\":{\"signals\":[{\"content\":{\"blockPath\":[\"ROMmodel_C4_C5/Model Outputs/IVD Rotation Readings/L5-S1 Moment-Rotation\"],\"dimensions\":[1,1],\"domain\":\"ROMmodel_C4_C5/Model Outputs/IVD Rotation Readings/L5-S1 Moment-Rotation\",\"lineColor\":\"#ff6929\",\"port\":1,\"sid\":[\"\"],\"signalID\":103,\"signalName\":\"In8\"},\"type\":\"RecordBlkView.Signal\",\"uuid\":\"e0e195aa-9f74-4331-a748-e761fe497069\"},{\"content\":{\"blockPath\":[\"ROMmodel_C4_C5/Model Outputs/IVD Rotation Readings/L5-S1 Moment-Rotation\"],\"dimensions\":[1,1],\"domain\":\"ROMmodel_C4_C5/Model Outputs/IVD Rotation Readings/L5-S1 Moment-Rotation\",\"lineColor\":\"#b746ff\",\"port\":2,\"sid\":[\"\"],\"signalID\":107,\"signalName\":\"L5-S1 FE Rot\"},\"type\":\"RecordBlkView.Signal\",\"uuid\":\"2c21c38b-a9ea-4718-b96f-d0a386546bed\"}]},\"type\":\"RecordBlkView.InputSignals\",\"uuid\":\"308917f2-3cf1-4730-94cf-50496bd68991\"}],\"packageUris\":[\"http://schema.mathworks.com/mf0/streamout_blocks/R2020a\"],\"version\":\"1.0\"}"  VisualizationBindings="{\"xyplotplugin\":{\"info\":[{\"series\":[],\"subplotID\":1}]}}"  CompatibilityTag=XY
  Radians to Degrees{,5..8} (5 alike) : simulink_extras/Transformations/Radians to Degrees
  Rotations : Scope  NumInputPorts=5
  Scope{2..3,7..10} (6 alike) : Scope
  L1-L2 FE Rot : Outport
  L2-L3 FE Rot : Outport  Port=2
  L3-L4 FE Rot : Outport  Port=3
  L4-L5 FE Rot : Outport  Port=4
  L5-S1 FE Rot : Outport  Port=5
  wires:
  Add10/1 -> Display6/1
  Gain8/1 -> L1-L2 FE Rot/1, Check L1-L2 Flexion/1, Add10/1, Display1/1, Rotations/1, L1-L2 Moment-Rotation/2, Scope10/1  "L1-L2 FE Rot"
  Add7/1 -> Gain6/1
  Radians to Degrees7/1 -> Add8/1
  Add3/1 -> Gain2/1
  Add6/1 -> Gain5/1
  Constant/1 -> Add3/2
  Gain5/1 -> Check L4-L5 Flexion/1, L4-L5 Moment-Rotation/2, L4-L5 FE Rot/1, Add10/4, Display4/1, Rotations/4, Scope7/1  "L4-L5 FE Rot"
  Gain2/1 -> Check L5-S1 Flexion/1, L5-S1 FE Rot/1, L5-S1 Moment-Rotation/2, Add10/5, Display5/1, Rotations/5, Scope2/1  "L5-S1 FE Rot"
  Radians in1/1 -> Radians to Degrees5/1
  Gain6/1 -> Check L3-L4 Flexion/1, Add10/3, Display3/1, Rotations/3, L3-L4 FE Rot/1, L3-L4 Moment-Rotation/2, Scope9/1  "L3-L4 FE Rot"
  Gain7/1 -> Check L2-L3 Flexion/1, L2-L3 FE Rot/1, L2-L3 Moment-Rotation/2, Add10/2, Display2/1, Rotations/2, Scope8/1  "L2-L3 FE Rot"
  Constant6/1 -> Add6/2
  In9/1 -> L2-L3 Moment-Rotation/1
  Radians to Degrees5/1 -> Add6/1
  Constant9/1 -> Add9/2
  Radians to Degrees6/1 -> Add7/1
  Constant8/1 -> Add8/2
  Add8/1 -> Gain7/1
  Radians to Degrees8/1 -> Add9/1
  Radians in3/1 -> Radians to Degrees7/1
  Constant7/1 -> Add7/2
  Add9/1 -> Gain8/1
  Radians to Degrees/1 -> Scope3/1, Add3/1
  Radians in/1 -> Radians to Degrees/1
  Radians in4/1 -> Radians to Degrees8/1
  Radians in2/1 -> Radians to Degrees6/1
  In7/1 -> L3-L4 Moment-Rotation/1
  In8/1 -> L5-S1 Moment-Rotation/1
  In5/1 -> L4-L5 Moment-Rotation/1
  In10/1 -> L1-L2 Moment-Rotation/1

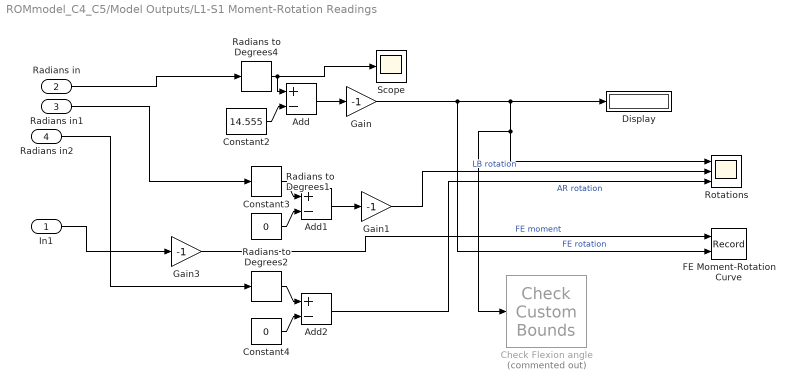
== system ROMmodel_C4_C5/Model Outputs/L1-S1 Moment-Rotation Readings ==
  In1 : Inport
  Radians in : Inport  Port=2
  Radians in1 : Inport  Port=3
  Radians in2 : Inport  Port=4
  Add : Sum  Inputs=+-
  Add1 : Sum  Inputs=+-
  Add2 : Sum  Inputs=+-
  Check Flexion angle : checkpacklib/Check Custom Bounds  Commented=on  InstantiateOnLoad=on  enabled=off  stopWhenAssertionFail=off  export=off  ViewDlgPos=[696 366 509 389]  OpenViewOnLoad=off  LaunchViewOnOpen=on  TimeUnits=seconds  EnableUpperBound=on  UpperBoundTimes=[0 1]  UpperBoundAmplitudes=[5 5];  EnableLowerBound=on  LowerBoundTimes=[0 1]  LowerBoundAmplitudes=[-5 -5];
      settings: LibrarySourceBlock=sdolib/Signal Constraints/Check Custom Bounds
  Constant2 : Constant  Value=14.555
  Constant3 : Constant  Value=0
  Constant4 : Constant  Value=0
  Display : Display
  FE Moment-Rotation Curve : Record  FileSettingsInternal="{\"entries\":[{\"content\":{\"excelSettings\":{\"content\":{},\"type\":\"Streamout.ExcelSettings\"}},\"type\":\"Streamout.FileSettingStorage\",\"uuid\":\"a0c54fad-ec13-4bb2-ae10-8f2629e36052\"}],\"packageUris\":[\"http://schema.mathworks.com/mf0/streamout_blocks/R2020a\"],\"version\":\"1.0\"}"  Layout=[1 1]  SignalsInternal="{\"entries\":[{\"content\":{},\"type\":\"RecordBlkView.InputSignals\",\"uuid\":\"39c741bb-febb-4010-b5af-191d4edcd744\"}],\"packageUris\":[\"http://schema.mathworks.com/mf0/streamout_blocks/R2020a\"],\"version\":\"1.0\"}"  VisualizationBindings="{\"xyplotplugin\":{\"info\":[{\"series\":[{\"bindingParametersList\":[{\"parameter\":\"X-Axis\",\"signalID\":111,\"signalName\":\"FE moment\"},{\"parameter\":\"Y-Axis\",\"signalID\":115,\"signalName\":\"FE rot\"}],\"seriesID\":64327}],\"subplotID\":1}]}}"  CompatibilityTag=XY
  Gain : Gain  Gain=-1
  Gain1 : Gain  Gain=-1
  Gain3 : Gain  Gain=-1
  Radians to Degrees1 : simulink_extras/Transformations/Radians to Degrees
  Radians to Degrees2 : simulink_extras/Transformations/Radians to Degrees
  Radians to Degrees4 : simulink_extras/Transformations/Radians to Degrees
  Rotations : Scope  NumInputPorts=3
  Scope : Scope
  wires:
  Add/1 -> Gain/1
  Radians to Degrees2/1 -> Add2/1
  Gain/1 -> Rotations/1, Check Flexion angle/1, Display/1, FE Moment-Rotation Curve/2  "FE rotation"
  Gain1/1 -> Rotations/2  "LB rotation"
  Radians to Degrees4/1 -> Add/1, Scope/1
  Constant4/1 -> Add2/2
  Add1/1 -> Gain1/1
  Radians to Degrees1/1 -> Add1/1
  Constant3/1 -> Add1/2
  Gain3/1 -> FE Moment-Rotation Curve/1  "FE moment"
  Constant2/1 -> Add/2
  Add2/1 -> Rotations/3  "AR rotation"
  Radians in/1 -> Radians to Degrees4/1
  Radians in2/1 -> Radians to Degrees2/1
  In1/1 -> Gain3/1
  Radians in1/1 -> Radians to Degrees1/1

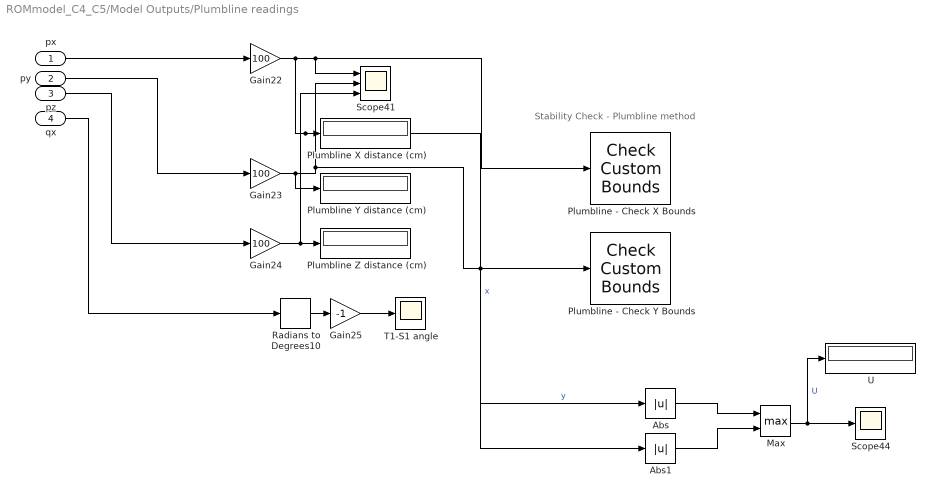
== system ROMmodel_C4_C5/Model Outputs/Plumbline readings ==
  note: Stability Check - Plumbline method
  px : Inport
  py : Inport  Port=2
  pz : Inport  Port=3
  qx : Inport  Port=4
  Abs : Abs  SaturateOnIntegerOverflow=off
  Abs1 : Abs  SaturateOnIntegerOverflow=off
  Gain22 : Gain  Gain=100
  Gain23 : Gain  Gain=100
  Gain24 : Gain  Gain=100
  Gain25 : Gain  Gain=-1
  Max : MinMax  Function=max  Inputs=2
  Plumbline - Check X Bounds : checkpacklib/Check Custom Bounds  InstantiateOnLoad=on  enabled=off  stopWhenAssertionFail=off  export=off  ViewDlgPos=[983.4 172.2 510.4 379.2]  OpenViewOnLoad=off  LaunchViewOnOpen=off  TimeUnits=seconds  EnableUpperBound=on  UpperBoundTimes=[0 1]  UpperBoundAmplitudes=[2 2];  EnableLowerBound=on  LowerBoundTimes=[0 1]  LowerBoundAmplitudes=[-2 -2]
      settings: LibrarySourceBlock=sdolib/Signal Constraints/Check Custom Bounds
  Plumbline - Check Y Bounds : checkpacklib/Check Custom Bounds  InstantiateOnLoad=on  enabled=off  stopWhenAssertionFail=off  export=off  ViewDlgPos=[509 222 510 390]  OpenViewOnLoad=off  LaunchViewOnOpen=off  TimeUnits=seconds  EnableUpperBound=on  UpperBoundTimes=[0 1]  UpperBoundAmplitudes=[2 2];  EnableLowerBound=on  LowerBoundTimes=[0 1]  LowerBoundAmplitudes=[-2 -2]
      settings: LibrarySourceBlock=sdolib/Signal Constraints/Check Custom Bounds
  Plumbline X distance (cm) : Display
  Plumbline Y distance (cm) : Display
  Plumbline Z distance (cm) : Display
  Radians to Degrees10 : simulink_extras/Transformations/Radians to Degrees
  Scope41 : Scope  NumInputPorts=3
  Scope44 : Scope
  T1-S1 angle : Scope
  U : Display
  wires:
  Gain22/1 -> Plumbline - Check X Bounds/1, Scope41/1, Abs1/1, Plumbline X distance (cm)/1  "x"
  Gain23/1 -> Scope41/2, Abs/1, Plumbline - Check Y Bounds/1, Plumbline Y distance (cm)/1  "y"
  Abs/1 -> Max/1
  Gain25/1 -> T1-S1 angle/1
  pz/1 -> Gain24/1
  qx/1 -> Radians to Degrees10/1
  px/1 -> Gain22/1
  Gain24/1 -> Plumbline Z distance (cm)/1, Scope41/3
  Max/1 -> U/1, Scope44/1  "U"
  Abs1/1 -> Max/2
  Radians to Degrees10/1 -> Gain25/1
  py/1 -> Gain23/1

== matlab function ROMmodel_C4_C5/Model Outputs/Cost readings/MATLAB Function ==
  data: Psoas input; SumofCubeofForces output; MF input; LongThor input; EO input; IO input; RA input; SumofMuscleStress output
function [SumofCubeofForces, SumofMuscleStress] = fcn(Psoas,MF,LongThor,EO,IO,RA)

SumofCubeofForces = (1e-6) * (Psoas^3 + MF^3 + LongThor^3 + EO^3 + IO^3 + RA^3) ;



% PCSA from Comparison of muscle forces and joint load from an optimization and EMG assisted lumbar spine model: Towards development of a hybrid approach
% Cholewicki 
% https://doi.org/10.1016/0021-9290(94)00065-C

PCSA_Psoas = 440; % mm^2
PCSA_MF = 1000 ;
PCSA_LongThor = 800 ;
PCSA_EO = 950 ;
PCSA_IO = 850 ;
PCSA_RA = 1000 ;

SumofMuscleStress = (Psoas/PCSA_Psoas) + (MF/PCSA_MF) + (LongThor/PCSA_LongThor) + (EO/PCSA_EO) + (IO/PCSA_IO) + (RA/PCSA_RA) ;  % MPa

== matlab function ROMmodel_C4_C5/Model Outputs/IVD Force Readings/MATLAB Function ==
  data: a input; SumofSquareofIVDForces output; b input; c input; d input; e input; f input; g input; h input; i input; j input
function SumofSquareofIVDForces = fcn(a,b,c,d,e,f,g,h,i,j)

SumofSquareofIVDForces = (1e-5) *(a^2 + b^2 + c^2 + d^2 + e^2 + f^2 + g^2 + h^2 + i^2 + j^2) ; % x 10e-5 N^2
